simulink model "Lab2_simulink_plc"  (R2023b) (continued)
== system Lab2_simulink_plc/PLC Controller Suite/__Logic/IR logic/IR sensor logic/__Logic == (continued)
      mask init:
      | block = gcb;
      | maskObj = Simulink.Mask.get(block);
      | maskObj.Display = sprintf('disp([''%s'' newline newline ''%s'']);', PLCBlockType, PLCOperandTag);
      | slplc.callbacks.initInstruction(block);
      settings: AttributesFormatString=%<PLCBlockType>: %<PLCOperandTag>
      mask Help: web([docroot '/plccoder/ref/ton' '.html']);
      mask DialogControl PLCBlockTitle Prompt: Timer On Delay (TON)
      mask Display: disp(['TON' newline newline 'medium_on']);
  TON5 : Subsystem  Priority=74  TreatAsAtomicUnit=on  mask{PLCOperandTag=medium_off, PLCTimerSampleTime=0.2, PLCBlockType=TON, PLCPOUType=stdFB, PLCOperandAccess=ReadWrite, PLCOperandExpression=xxx_PLC_VAR_medium_off}
      mask prompts: PLCOperandTag "Timer Tag:"; PLCTimerSampleTime "Timer Sample Time:"
      mask init:
      | block = gcb;
      | maskObj = Simulink.Mask.get(block);
      | maskObj.Display = sprintf('disp([''%s'' newline newline ''%s'']);', PLCBlockType, PLCOperandTag);
      | slplc.callbacks.initInstruction(block);
      settings: AttributesFormatString=%<PLCBlockType>: %<PLCOperandTag>
      mask Help: web([docroot '/plccoder/ref/ton' '.html']);
      mask DialogControl PLCBlockTitle Prompt: Timer On Delay (TON)
      mask Display: disp(['TON' newline newline 'medium_off']);
  Up to Parent POU : Subsystem  DisableCoverage=on  TreatAsAtomicUnit=on  mask{PLCBlockType=OpenParentPOU}
      settings: OpenFcn=slplc.callbacks.openParentPOUBlock(gcb);
      mask Help: web([docroot '/plccoder/ref/uptoparentpou' '.html']);
      mask DialogControl PLCBlockTitle Prompt: Add Single Rung
  Variable Read : Subsystem  Priority=34  TreatAsAtomicUnit=on  mask{PLCOperandTag=IR_value_copy, PLCBlockType=VariableRead, PLCOperandAccess=Read, PLCPOUType=VariableRead, PLCOperandExpression=xxx_PLC_VAR_IR_value_copy}
      mask prompts: PLCOperandTag "Data Tag:"
      mask init:
      | block = gcb;
      | maskObj = Simulink.Mask.get(block);
      | maskObj.Display = sprintf('disp(''%s'');', PLCOperandTag);
      | slplc.callbacks.initInstruction(block);
      mask Help: web([docroot '/plccoder/ref/variableread' '.html']);
      mask DialogControl PLCBlockTitle Prompt: Data Tag Read
      mask Display: disp('IR_value_copy');
  Variable Read1 : Subsystem  Priority=7  TreatAsAtomicUnit=on  mask{PLCOperandTag=IR_value_copy, PLCBlockType=VariableRead, PLCOperandAccess=Read, PLCPOUType=VariableRead, PLCOperandExpression=xxx_PLC_VAR_IR_value_copy}
      mask prompts: PLCOperandTag "Data Tag:"
      mask init:
      | block = gcb;
      | maskObj = Simulink.Mask.get(block);
      | maskObj.Display = sprintf('disp(''%s'');', PLCOperandTag);
      | slplc.callbacks.initInstruction(block);
      mask Help: web([docroot '/plccoder/ref/variableread' '.html']);
      mask DialogControl PLCBlockTitle Prompt: Data Tag Read
      mask Display: disp('IR_value_copy');
  Variable Read10 : Subsystem  Priority=2  TreatAsAtomicUnit=on  mask{PLCOperandTag=IR_value_copy, PLCBlockType=VariableRead, PLCOperandAccess=Read, PLCPOUType=VariableRead, PLCOperandExpression=xxx_PLC_VAR_IR_value_copy}
      mask prompts: PLCOperandTag "Data Tag:"
      mask init:
      | block = gcb;
      | maskObj = Simulink.Mask.get(block);
      | maskObj.Display = sprintf('disp(''%s'');', PLCOperandTag);
      | slplc.callbacks.initInstruction(block);
      mask Help: web([docroot '/plccoder/ref/variableread' '.html']);
      mask DialogControl PLCBlockTitle Prompt: Data Tag Read
      mask Display: disp('IR_value_copy');
  Variable Read11 : Subsystem  Priority=24  TreatAsAtomicUnit=on  mask{PLCOperandTag=half_constant, PLCBlockType=VariableRead, PLCOperandAccess=Read, PLCPOUType=VariableRead, PLCOperandExpression=xxx_PLC_VAR_half_constant}
      mask prompts: PLCOperandTag "Data Tag:"
      mask init:
      | block = gcb;
      | maskObj = Simulink.Mask.get(block);
      | maskObj.Display = sprintf('disp(''%s'');', PLCOperandTag);
      | slplc.callbacks.initInstruction(block);
      mask Help: web([docroot '/plccoder/ref/variableread' '.html']);
      mask DialogControl PLCBlockTitle Prompt: Data Tag Read
      mask Display: disp('half_constant');
  Variable Read12 : Subsystem  Priority=16  TreatAsAtomicUnit=on  mask{PLCOperandTag=IR_value_copy, PLCBlockType=VariableRead, PLCOperandAccess=Read, PLCPOUType=VariableRead, PLCOperandExpression=xxx_PLC_VAR_IR_value_copy}
      mask prompts: PLCOperandTag "Data Tag:"
      mask init:
      | block = gcb;
      | maskObj = Simulink.Mask.get(block);
      | maskObj.Display = sprintf('disp(''%s'');', PLCOperandTag);
      | slplc.callbacks.initInstruction(block);
      mask Help: web([docroot '/plccoder/ref/variableread' '.html']);
      mask DialogControl PLCBlockTitle Prompt: Data Tag Read
      mask Display: disp('IR_value_copy');
  Variable Read13 : Subsystem  Priority=17  TreatAsAtomicUnit=on  mask{PLCOperandTag=close_constant, PLCBlockType=VariableRead, PLCOperandAccess=Read, PLCPOUType=VariableRead, PLCOperandExpression=xxx_PLC_VAR_close_constant}
      mask prompts: PLCOperandTag "Data Tag:"
      mask init:
      | block = gcb;
      | maskObj = Simulink.Mask.get(block);
      | maskObj.Display = sprintf('disp(''%s'');', PLCOperandTag);
      | slplc.callbacks.initInstruction(block);
      mask Help: web([docroot '/plccoder/ref/variableread' '.html']);
      mask DialogControl PLCBlockTitle Prompt: Data Tag Read
      mask Display: disp('close_constant');
  Variable Read14 : Subsystem  Priority=40  TreatAsAtomicUnit=on  mask{PLCOperandTag=duty_cycle, PLCBlockType=VariableRead, PLCOperandAccess=Read, PLCPOUType=VariableRead, PLCOperandExpression=xxx_PLC_VAR_duty_cycle}
      mask prompts: PLCOperandTag "Data Tag:"
      mask init:
      | block = gcb;
      | maskObj = Simulink.Mask.get(block);
      | maskObj.Display = sprintf('disp(''%s'');', PLCOperandTag);
      | slplc.callbacks.initInstruction(block);
      mask Help: web([docroot '/plccoder/ref/variableread' '.html']);
      mask DialogControl PLCBlockTitle Prompt: Data Tag Read
      mask Display: disp('duty_cycle');
  Variable Read15 : Subsystem  Priority=47  TreatAsAtomicUnit=on  mask{PLCOperandTag=duty_cycle, PLCBlockType=VariableRead, PLCOperandAccess=Read, PLCPOUType=VariableRead, PLCOperandExpression=xxx_PLC_VAR_duty_cycle}
      mask prompts: PLCOperandTag "Data Tag:"
      mask init:
      | block = gcb;
      | maskObj = Simulink.Mask.get(block);
      | maskObj.Display = sprintf('disp(''%s'');', PLCOperandTag);
      | slplc.callbacks.initInstruction(block);
      mask Help: web([docroot '/plccoder/ref/variableread' '.html']);
      mask DialogControl PLCBlockTitle Prompt: Data Tag Read
      mask Display: disp('duty_cycle');
  Variable Read16 : Subsystem  Priority=55  TreatAsAtomicUnit=on  mask{PLCOperandTag=green_off, PLCBlockType=VariableRead, PLCOperandAccess=Read, PLCPOUType=VariableRead, PLCOperandExpression=xxx_PLC_VAR_green_off}
      mask prompts: PLCOperandTag "Data Tag:"
      mask init:
      | block = gcb;
      | maskObj = Simulink.Mask.get(block);
      | maskObj.Display = sprintf('disp(''%s'');', PLCOperandTag);
      | slplc.callbacks.initInstruction(block);
      mask Help: web([docroot '/plccoder/ref/variableread' '.html']);
      mask DialogControl PLCBlockTitle Prompt: Data Tag Read
      mask Display: disp('green_off');
  Variable Read17 : Subsystem  Priority=63  TreatAsAtomicUnit=on  mask{PLCOperandTag=duty_cycle, PLCBlockType=VariableRead, PLCOperandAccess=Read, PLCPOUType=VariableRead, PLCOperandExpression=xxx_PLC_VAR_duty_cycle}
      mask prompts: PLCOperandTag "Data Tag:"
      mask init:
      | block = gcb;
      | maskObj = Simulink.Mask.get(block);
      | maskObj.Display = sprintf('disp(''%s'');', PLCOperandTag);
      | slplc.callbacks.initInstruction(block);
      mask Help: web([docroot '/plccoder/ref/variableread' '.html']);
      mask DialogControl PLCBlockTitle Prompt: Data Tag Read
      mask Display: disp('duty_cycle');
  Variable Read18 : Subsystem  Priority=79  TreatAsAtomicUnit=on  mask{PLCOperandTag=duty_cycle, PLCBlockType=VariableRead, PLCOperandAccess=Read, PLCPOUType=VariableRead, PLCOperandExpression=xxx_PLC_VAR_duty_cycle}
      mask prompts: PLCOperandTag "Data Tag:"
      mask init:
      | block = gcb;
      | maskObj = Simulink.Mask.get(block);
      | maskObj.Display = sprintf('disp(''%s'');', PLCOperandTag);
      | slplc.callbacks.initInstruction(block);
      mask Help: web([docroot '/plccoder/ref/variableread' '.html']);
      mask DialogControl PLCBlockTitle Prompt: Data Tag Read
      mask Display: disp('duty_cycle');
  Variable Read19 : Subsystem  Priority=71  TreatAsAtomicUnit=on  mask{PLCOperandTag=green_off, PLCBlockType=VariableRead, PLCOperandAccess=Read, PLCPOUType=VariableRead, PLCOperandExpression=xxx_PLC_VAR_green_off}
      mask prompts: PLCOperandTag "Data Tag:"
      mask init:
      | block = gcb;
      | maskObj = Simulink.Mask.get(block);
      | maskObj.Display = sprintf('disp(''%s'');', PLCOperandTag);
      | slplc.callbacks.initInstruction(block);
      mask Help: web([docroot '/plccoder/ref/variableread' '.html']);
      mask DialogControl PLCBlockTitle Prompt: Data Tag Read
      mask Display: disp('green_off');
  Variable Read2 : Subsystem  Priority=35  TreatAsAtomicUnit=on  mask{PLCOperandTag=far_constant, PLCBlockType=VariableRead, PLCOperandAccess=Read, PLCPOUType=VariableRead, PLCOperandExpression=xxx_PLC_VAR_far_constant}
      mask prompts: PLCOperandTag "Data Tag:"
      mask init:
      | block = gcb;
      | maskObj = Simulink.Mask.get(block);
      | maskObj.Display = sprintf('disp(''%s'');', PLCOperandTag);
      | slplc.callbacks.initInstruction(block);
      mask Help: web([docroot '/plccoder/ref/variableread' '.html']);
      mask DialogControl PLCBlockTitle Prompt: Data Tag Read
      mask Display: disp('far_constant');
  Variable Read20 : Subsystem  Priority=87  TreatAsAtomicUnit=on  mask{PLCOperandTag=green_off, PLCBlockType=VariableRead, PLCOperandAccess=Read, PLCPOUType=VariableRead, PLCOperandExpression=xxx_PLC_VAR_green_off}
      mask prompts: PLCOperandTag "Data Tag:"
      mask init:
      | block = gcb;
      | maskObj = Simulink.Mask.get(block);
      | maskObj.Display = sprintf('disp(''%s'');', PLCOperandTag);
      | slplc.callbacks.initInstruction(block);
      mask Help: web([docroot '/plccoder/ref/variableread' '.html']);
      mask DialogControl PLCBlockTitle Prompt: Data Tag Read
      mask Display: disp('green_off');
  Variable Read3 : Subsystem  Priority=8  TreatAsAtomicUnit=on  mask{PLCOperandTag=scope_constant, PLCBlockType=VariableRead, PLCOperandAccess=Read, PLCPOUType=VariableRead, PLCOperandExpression=xxx_PLC_VAR_scope_constant}
      mask prompts: PLCOperandTag "Data Tag:"
      mask init:
      | block = gcb;
      | maskObj = Simulink.Mask.get(block);
      | maskObj.Display = sprintf('disp(''%s'');', PLCOperandTag);
      | slplc.callbacks.initInstruction(block);
      mask Help: web([docroot '/plccoder/ref/variableread' '.html']);
      mask DialogControl PLCBlockTitle Prompt: Data Tag Read
      mask Display: disp('scope_constant');
  Variable Read4 : Subsystem  Priority=13  TreatAsAtomicUnit=on  mask{PLCOperandTag=IR_value_copy, PLCBlockType=VariableRead, PLCOperandAccess=Read, PLCPOUType=VariableRead, PLCOperandExpression=xxx_PLC_VAR_IR_value_copy}
      mask prompts: PLCOperandTag "Data Tag:"
      mask init:
      | block = gcb;
      | maskObj = Simulink.Mask.get(block);
      | maskObj.Display = sprintf('disp(''%s'');', PLCOperandTag);
      | slplc.callbacks.initInstruction(block);
      mask Help: web([docroot '/plccoder/ref/variableread' '.html']);
      mask DialogControl PLCBlockTitle Prompt: Data Tag Read
      mask Display: disp('IR_value_copy');
  Variable Read5 : Subsystem  Priority=14  TreatAsAtomicUnit=on  mask{PLCOperandTag=half_constant, PLCBlockType=VariableRead, PLCOperandAccess=Read, PLCPOUType=VariableRead, PLCOperandExpression=xxx_PLC_VAR_half_constant}
      mask prompts: PLCOperandTag "Data Tag:"
      mask init:
      | block = gcb;
      | maskObj = Simulink.Mask.get(block);
      | maskObj.Display = sprintf('disp(''%s'');', PLCOperandTag);
      | slplc.callbacks.initInstruction(block);
      mask Help: web([docroot '/plccoder/ref/variableread' '.html']);
      mask DialogControl PLCBlockTitle Prompt: Data Tag Read
      mask Display: disp('half_constant');
  Variable Read6 : Subsystem  Priority=23  TreatAsAtomicUnit=on  mask{PLCOperandTag=IR_value_copy, PLCBlockType=VariableRead, PLCOperandAccess=Read, PLCPOUType=VariableRead, PLCOperandExpression=xxx_PLC_VAR_IR_value_copy}
      mask prompts: PLCOperandTag "Data Tag:"
      mask init:
      | block = gcb;
      | maskObj = Simulink.Mask.get(block);
      | maskObj.Display = sprintf('disp(''%s'');', PLCOperandTag);
      | slplc.callbacks.initInstruction(block);
      mask Help: web([docroot '/plccoder/ref/variableread' '.html']);
      mask DialogControl PLCBlockTitle Prompt: Data Tag Read
      mask Display: disp('IR_value_copy');
  Variable Read7 : Subsystem  Priority=26  TreatAsAtomicUnit=on  mask{PLCOperandTag=IR_value_copy, PLCBlockType=VariableRead, PLCOperandAccess=Read, PLCPOUType=VariableRead, PLCOperandExpression=xxx_PLC_VAR_IR_value_copy}
      mask prompts: PLCOperandTag "Data Tag:"
      mask init:
      | block = gcb;
      | maskObj = Simulink.Mask.get(block);
      | maskObj.Display = sprintf('disp(''%s'');', PLCOperandTag);
      | slplc.callbacks.initInstruction(block);
      mask Help: web([docroot '/plccoder/ref/variableread' '.html']);
      mask DialogControl PLCBlockTitle Prompt: Data Tag Read
      mask Display: disp('IR_value_copy');
  Variable Read8 : Subsystem  Priority=27  TreatAsAtomicUnit=on  mask{PLCOperandTag=far_constant, PLCBlockType=VariableRead, PLCOperandAccess=Read, PLCPOUType=VariableRead, PLCOperandExpression=xxx_PLC_VAR_far_constant}
      mask prompts: PLCOperandTag "Data Tag:"
      mask init:
      | block = gcb;
      | maskObj = Simulink.Mask.get(block);
      | maskObj.Display = sprintf('disp(''%s'');', PLCOperandTag);
      | slplc.callbacks.initInstruction(block);
      mask Help: web([docroot '/plccoder/ref/variableread' '.html']);
      mask DialogControl PLCBlockTitle Prompt: Data Tag Read
      mask Display: disp('far_constant');
  Variable Read9 : Subsystem  Priority=3  TreatAsAtomicUnit=on  mask{PLCOperandTag=constant, PLCBlockType=VariableRead, PLCOperandAccess=Read, PLCPOUType=VariableRead, PLCOperandExpression=xxx_PLC_VAR_constant}
      mask prompts: PLCOperandTag "Data Tag:"
      mask init:
      | block = gcb;
      | maskObj = Simulink.Mask.get(block);
      | maskObj.Display = sprintf('disp(''%s'');', PLCOperandTag);
      | slplc.callbacks.initInstruction(block);
      mask Help: web([docroot '/plccoder/ref/variableread' '.html']);
      mask DialogControl PLCBlockTitle Prompt: Data Tag Read
      mask Display: disp('constant');
  Variable Write : Subsystem  Priority=5  TreatAsAtomicUnit=on  mask{PLCOperandTag=duty_cycle, PLCBlockType=VariableWrite, PLCOperandAccess=Write, PLCPOUType=VariableWrite, PLCOperandExpression=xxx_PLC_VAR_duty_cycle}
      mask prompts: PLCOperandTag "Data Tag:"
      mask init: same as Lab2_simulink_plc/PLC Controller Suite/__Logic/Fukt logic/Program/__Logic/Variable Write
      mask Help: web([docroot '/plccoder/ref/variablewrite' '.html']);
      mask DialogControl PLCBlockTitle Prompt: Data Tag Write
      mask Display: disp('duty_cycle');
  Variable Write1 : Subsystem  Priority=42  TreatAsAtomicUnit=on  mask{PLCOperandTag=GreenLed, PLCBlockType=VariableWrite, PLCOperandAccess=Write, PLCPOUType=VariableWrite, PLCOperandExpression=xxx_PLC_VAR_GreenLed}
      mask prompts: PLCOperandTag "Data Tag:"
      mask init: same as Lab2_simulink_plc/PLC Controller Suite/__Logic/Fukt logic/Program/__Logic/Variable Write
      mask Help: web([docroot '/plccoder/ref/variablewrite' '.html']);
      mask DialogControl PLCBlockTitle Prompt: Data Tag Write
      mask Display: disp('GreenLed');
  Variable Write2 : Subsystem  Priority=49  TreatAsAtomicUnit=on  mask{PLCOperandTag=GreenLed, PLCBlockType=VariableWrite, PLCOperandAccess=Write, PLCPOUType=VariableWrite, PLCOperandExpression=xxx_PLC_VAR_GreenLed}
      mask prompts: PLCOperandTag "Data Tag:"
      mask init: same as Lab2_simulink_plc/PLC Controller Suite/__Logic/Fukt logic/Program/__Logic/Variable Write
      mask Help: web([docroot '/plccoder/ref/variablewrite' '.html']);
      mask DialogControl PLCBlockTitle Prompt: Data Tag Write
      mask Display: disp('GreenLed');
  Variable Write3 : Subsystem  Priority=57  TreatAsAtomicUnit=on  mask{PLCOperandTag=GreenLed, PLCBlockType=VariableWrite, PLCOperandAccess=Write, PLCPOUType=VariableWrite, PLCOperandExpression=xxx_PLC_VAR_GreenLed}
      mask prompts: PLCOperandTag "Data Tag:"
      mask init: same as Lab2_simulink_plc/PLC Controller Suite/__Logic/Fukt logic/Program/__Logic/Variable Write
      mask Help: web([docroot '/plccoder/ref/variablewrite' '.html']);
      mask DialogControl PLCBlockTitle Prompt: Data Tag Write
      mask Display: disp('GreenLed');
  Variable Write4 : Subsystem  Priority=65  TreatAsAtomicUnit=on  mask{PLCOperandTag=GreenLed, PLCBlockType=VariableWrite, PLCOperandAccess=Write, PLCPOUType=VariableWrite, PLCOperandExpression=xxx_PLC_VAR_GreenLed}
      mask prompts: PLCOperandTag "Data Tag:"
      mask init: same as Lab2_simulink_plc/PLC Controller Suite/__Logic/Fukt logic/Program/__Logic/Variable Write
      mask Help: web([docroot '/plccoder/ref/variablewrite' '.html']);
      mask DialogControl PLCBlockTitle Prompt: Data Tag Write
      mask Display: disp('GreenLed');
  Variable Write5 : Subsystem  Priority=81  TreatAsAtomicUnit=on  mask{PLCOperandTag=GreenLed, PLCBlockType=VariableWrite, PLCOperandAccess=Write, PLCPOUType=VariableWrite, PLCOperandExpression=xxx_PLC_VAR_GreenLed}
      mask prompts: PLCOperandTag "Data Tag:"
      mask init: same as Lab2_simulink_plc/PLC Controller Suite/__Logic/Fukt logic/Program/__Logic/Variable Write
      mask Help: web([docroot '/plccoder/ref/variablewrite' '.html']);
      mask DialogControl PLCBlockTitle Prompt: Data Tag Write
      mask Display: disp('GreenLed');
  Variable Write6 : Subsystem  Priority=73  TreatAsAtomicUnit=on  mask{PLCOperandTag=GreenLed, PLCBlockType=VariableWrite, PLCOperandAccess=Write, PLCPOUType=VariableWrite, PLCOperandExpression=xxx_PLC_VAR_GreenLed}
      mask prompts: PLCOperandTag "Data Tag:"
      mask init: same as Lab2_simulink_plc/PLC Controller Suite/__Logic/Fukt logic/Program/__Logic/Variable Write
      mask Help: web([docroot '/plccoder/ref/variablewrite' '.html']);
      mask DialogControl PLCBlockTitle Prompt: Data Tag Write
      mask Display: disp('GreenLed');
  Variable Write7 : Subsystem  Priority=89  TreatAsAtomicUnit=on  mask{PLCOperandTag=GreenLed, PLCBlockType=VariableWrite, PLCOperandAccess=Write, PLCPOUType=VariableWrite, PLCOperandExpression=xxx_PLC_VAR_GreenLed}
      mask prompts: PLCOperandTag "Data Tag:"
      mask init: same as Lab2_simulink_plc/PLC Controller Suite/__Logic/Fukt logic/Program/__Logic/Variable Write
      mask Help: web([docroot '/plccoder/ref/variablewrite' '.html']);
      mask DialogControl PLCBlockTitle Prompt: Data Tag Write
      mask Display: disp('GreenLed');
  XIC : Subsystem  Priority=86  TreatAsAtomicUnit=on  mask{PLCOperandTag=slow_on.DN, PLCBlockType=XIC, PLCPOUType=Contact, PLCOperandExpression=xxx_PLC_VAR_slow_on.DN}
      mask prompts: PLCOperandTag "Operand Tag:"
      mask init:
      | slplc.callbacks.initInstruction(gcb);
      settings: AttributesFormatString=%<PLCOperandTag>
      mask Help: web([docroot '/plccoder/ref/xic' '.html']);
      mask DialogControl PLCBlockTitle Prompt: Examine If Closed (XIC)
  XIC1 : Subsystem  Priority=39  TreatAsAtomicUnit=on  mask{PLCOperandTag=out_of_scope, PLCBlockType=XIC, PLCPOUType=Contact, PLCOperandExpression=xxx_PLC_VAR_out_of_scope}
      mask prompts: PLCOperandTag "Operand Tag:"
      mask init:
      | slplc.callbacks.initInstruction(gcb);
      settings: AttributesFormatString=%<PLCOperandTag>
      mask Help: web([docroot '/plccoder/ref/xic' '.html']);
      mask DialogControl PLCBlockTitle Prompt: Examine If Closed (XIC)
  XIC2 : Subsystem  Priority=53  TreatAsAtomicUnit=on  mask{PLCOperandTag=close, PLCBlockType=XIC, PLCPOUType=Contact, PLCOperandExpression=xxx_PLC_VAR_close}
      mask prompts: PLCOperandTag "Operand Tag:"
      mask init:
      | slplc.callbacks.initInstruction(gcb);
      settings: AttributesFormatString=%<PLCOperandTag>
      mask Help: web([docroot '/plccoder/ref/xic' '.html']);
      mask DialogControl PLCBlockTitle Prompt: Examine If Closed (XIC)
  XIC3 : Subsystem  Priority=61  TreatAsAtomicUnit=on  mask{PLCOperandTag=half, PLCBlockType=XIC, PLCPOUType=Contact, PLCOperandExpression=xxx_PLC_VAR_half}
      mask prompts: PLCOperandTag "Operand Tag:"
      mask init:
      | slplc.callbacks.initInstruction(gcb);
      settings: AttributesFormatString=%<PLCOperandTag>
      mask Help: web([docroot '/plccoder/ref/xic' '.html']);
      mask DialogControl PLCBlockTitle Prompt: Examine If Closed (XIC)
  XIC4 : Subsystem  Priority=54  TreatAsAtomicUnit=on  mask{PLCOperandTag=fast_on.DN, PLCBlockType=XIC, PLCPOUType=Contact, PLCOperandExpression=xxx_PLC_VAR_fast_on.DN}
      mask prompts: PLCOperandTag "Operand Tag:"
      mask init:
      | slplc.callbacks.initInstruction(gcb);
      settings: AttributesFormatString=%<PLCOperandTag>
      mask Help: web([docroot '/plccoder/ref/xic' '.html']);
      mask DialogControl PLCBlockTitle Prompt: Examine If Closed (XIC)
  XIC5 : Subsystem  Priority=70  TreatAsAtomicUnit=on  mask{PLCOperandTag=medium_on.DN, PLCBlockType=XIC, PLCPOUType=Contact, PLCOperandExpression=xxx_PLC_VAR_medium_on.DN}
      mask prompts: PLCOperandTag "Operand Tag:"
      mask init:
      | slplc.callbacks.initInstruction(gcb);
      settings: AttributesFormatString=%<PLCOperandTag>
      mask Help: web([docroot '/plccoder/ref/xic' '.html']);
      mask DialogControl PLCBlockTitle Prompt: Examine If Closed (XIC)
  XIC6 : Subsystem  Priority=45  TreatAsAtomicUnit=on  mask{PLCOperandTag=close, PLCBlockType=XIC, PLCPOUType=Contact, PLCOperandExpression=xxx_PLC_VAR_close}
      mask prompts: PLCOperandTag "Operand Tag:"
      mask init:
      | slplc.callbacks.initInstruction(gcb);
      settings: AttributesFormatString=%<PLCOperandTag>
      mask Help: web([docroot '/plccoder/ref/xic' '.html']);
      mask DialogControl PLCBlockTitle Prompt: Examine If Closed (XIC)
  XIC7 : Subsystem  Priority=69  TreatAsAtomicUnit=on  mask{PLCOperandTag=half, PLCBlockType=XIC, PLCPOUType=Contact, PLCOperandExpression=xxx_PLC_VAR_half}
      mask prompts: PLCOperandTag "Operand Tag:"
      mask init:
      | slplc.callbacks.initInstruction(gcb);
      settings: AttributesFormatString=%<PLCOperandTag>
      mask Help: web([docroot '/plccoder/ref/xic' '.html']);
      mask DialogControl PLCBlockTitle Prompt: Examine If Closed (XIC)
  XIC8 : Subsystem  Priority=77  TreatAsAtomicUnit=on  mask{PLCOperandTag=far, PLCBlockType=XIC, PLCPOUType=Contact, PLCOperandExpression=xxx_PLC_VAR_far}
      mask prompts: PLCOperandTag "Operand Tag:"
      mask init:
      | slplc.callbacks.initInstruction(gcb);
      settings: AttributesFormatString=%<PLCOperandTag>
      mask Help: web([docroot '/plccoder/ref/xic' '.html']);
      mask DialogControl PLCBlockTitle Prompt: Examine If Closed (XIC)
  XIC9 : Subsystem  Priority=85  TreatAsAtomicUnit=on  mask{PLCOperandTag=far, PLCBlockType=XIC, PLCPOUType=Contact, PLCOperandExpression=xxx_PLC_VAR_far}
      mask prompts: PLCOperandTag "Operand Tag:"
      mask init:
      | slplc.callbacks.initInstruction(gcb);
      settings: AttributesFormatString=%<PLCOperandTag>
      mask Help: web([docroot '/plccoder/ref/xic' '.html']);
      mask DialogControl PLCBlockTitle Prompt: Examine If Closed (XIC)
  XIO : Subsystem  Priority=78  TreatAsAtomicUnit=on  mask{PLCOperandExpression=xxx_PLC_VAR_slow_off.DN, PLCOperandTag=slow_off.DN, PLCBlockType=XIO, PLCPOUType=Contact}
      mask prompts: PLCOperandTag "Operand Tag:"
      mask init:
      | slplc.callbacks.initInstruction(gcb);
      settings: AttributesFormatString=%<PLCOperandTag>
      mask Help: web([docroot '/plccoder/ref/xio' '.html']);
      mask DialogControl PLCBlockTitle Prompt: Examine If Open (XIO)
  XIO1 : Subsystem  Priority=31  TreatAsAtomicUnit=on  mask{PLCOperandExpression=xxx_PLC_VAR_out_of_scope, PLCOperandTag=out_of_scope, PLCBlockType=XIO, PLCPOUType=Contact}
      mask prompts: PLCOperandTag "Operand Tag:"
      mask init:
      | slplc.callbacks.initInstruction(gcb);
      settings: AttributesFormatString=%<PLCOperandTag>
      mask Help: web([docroot '/plccoder/ref/xio' '.html']);
      mask DialogControl PLCBlockTitle Prompt: Examine If Open (XIO)
  XIO10 : Subsystem  Priority=76  TreatAsAtomicUnit=on  mask{PLCOperandExpression=xxx_PLC_VAR_out_of_scope, PLCOperandTag=out_of_scope, PLCBlockType=XIO, PLCPOUType=Contact}
      mask prompts: PLCOperandTag "Operand Tag:"
      mask init:
      | slplc.callbacks.initInstruction(gcb);
      settings: AttributesFormatString=%<PLCOperandTag>
      mask Help: web([docroot '/plccoder/ref/xio' '.html']);
      mask DialogControl PLCBlockTitle Prompt: Examine If Open (XIO)
  XIO11 : Subsystem  Priority=22  TreatAsAtomicUnit=on  mask{PLCOperandExpression=xxx_PLC_VAR_out_of_scope, PLCOperandTag=out_of_scope, PLCBlockType=XIO, PLCPOUType=Contact}
      mask prompts: PLCOperandTag "Operand Tag:"
      mask init:
      | slplc.callbacks.initInstruction(gcb);
      settings: AttributesFormatString=%<PLCOperandTag>
      mask Help: web([docroot '/plccoder/ref/xio' '.html']);
      mask DialogControl PLCBlockTitle Prompt: Examine If Open (XIO)
  XIO12 : Subsystem  Priority=21  TreatAsAtomicUnit=on  mask{PLCOperandExpression=xxx_PLC_VAR_close, PLCOperandTag=close, PLCBlockType=XIO, PLCPOUType=Contact}
      mask prompts: PLCOperandTag "Operand Tag:"
      mask init:
      | slplc.callbacks.initInstruction(gcb);
      settings: AttributesFormatString=%<PLCOperandTag>
      mask Help: web([docroot '/plccoder/ref/xio' '.html']);
      mask DialogControl PLCBlockTitle Prompt: Examine If Open (XIO)
  XIO13 : Subsystem  Priority=32  TreatAsAtomicUnit=on  mask{PLCOperandExpression=xxx_PLC_VAR_close, PLCOperandTag=close, PLCBlockType=XIO, PLCPOUType=Contact}
      mask prompts: PLCOperandTag "Operand Tag:"
      mask init:
      | slplc.callbacks.initInstruction(gcb);
      settings: AttributesFormatString=%<PLCOperandTag>
      mask Help: web([docroot '/plccoder/ref/xio' '.html']);
      mask DialogControl PLCBlockTitle Prompt: Examine If Open (XIO)
  XIO14 : Subsystem  Priority=33  TreatAsAtomicUnit=on  mask{PLCOperandExpression=xxx_PLC_VAR_half, PLCOperandTag=half, PLCBlockType=XIO, PLCPOUType=Contact}
      mask prompts: PLCOperandTag "Operand Tag:"
      mask init:
      | slplc.callbacks.initInstruction(gcb);
      settings: AttributesFormatString=%<PLCOperandTag>
      mask Help: web([docroot '/plccoder/ref/xio' '.html']);
      mask DialogControl PLCBlockTitle Prompt: Examine If Open (XIO)
  XIO2 : Subsystem  Priority=12  TreatAsAtomicUnit=on  mask{PLCOperandExpression=xxx_PLC_VAR_out_of_scope, PLCOperandTag=out_of_scope, PLCBlockType=XIO, PLCPOUType=Contact}
      mask prompts: PLCOperandTag "Operand Tag:"
      mask init:
      | slplc.callbacks.initInstruction(gcb);
      settings: AttributesFormatString=%<PLCOperandTag>
      mask Help: web([docroot '/plccoder/ref/xio' '.html']);
      mask DialogControl PLCBlockTitle Prompt: Examine If Open (XIO)
  XIO3 : Subsystem  Priority=52  TreatAsAtomicUnit=on  mask{PLCOperandExpression=xxx_PLC_VAR_out_of_scope, PLCOperandTag=out_of_scope, PLCBlockType=XIO, PLCPOUType=Contact}
      mask prompts: PLCOperandTag "Operand Tag:"
      mask init:
      | slplc.callbacks.initInstruction(gcb);
      settings: AttributesFormatString=%<PLCOperandTag>
      mask Help: web([docroot '/plccoder/ref/xio' '.html']);
      mask DialogControl PLCBlockTitle Prompt: Examine If Open (XIO)
  XIO4 : Subsystem  Priority=60  TreatAsAtomicUnit=on  mask{PLCOperandExpression=xxx_PLC_VAR_out_of_scope, PLCOperandTag=out_of_scope, PLCBlockType=XIO, PLCPOUType=Contact}
      mask prompts: PLCOperandTag "Operand Tag:"
      mask init:
      | slplc.callbacks.initInstruction(gcb);
      settings: AttributesFormatString=%<PLCOperandTag>
      mask Help: web([docroot '/plccoder/ref/xio' '.html']);
      mask DialogControl PLCBlockTitle Prompt: Examine If Open (XIO)
  XIO5 : Subsystem  Priority=84  TreatAsAtomicUnit=on  mask{PLCOperandExpression=xxx_PLC_VAR_out_of_scope, PLCOperandTag=out_of_scope, PLCBlockType=XIO, PLCPOUType=Contact}
      mask prompts: PLCOperandTag "Operand Tag:"
      mask init:
      | slplc.callbacks.initInstruction(gcb);
      settings: AttributesFormatString=%<PLCOperandTag>
      mask Help: web([docroot '/plccoder/ref/xio' '.html']);
      mask DialogControl PLCBlockTitle Prompt: Examine If Open (XIO)
  XIO6 : Subsystem  Priority=46  TreatAsAtomicUnit=on  mask{PLCOperandExpression=xxx_PLC_VAR_fast_off.DN, PLCOperandTag=fast_off.DN, PLCBlockType=XIO, PLCPOUType=Contact}
      mask prompts: PLCOperandTag "Operand Tag:"
      mask init:
      | slplc.callbacks.initInstruction(gcb);
      settings: AttributesFormatString=%<PLCOperandTag>
      mask Help: web([docroot '/plccoder/ref/xio' '.html']);
      mask DialogControl PLCBlockTitle Prompt: Examine If Open (XIO)
  XIO7 : Subsystem  Priority=62  TreatAsAtomicUnit=on  mask{PLCOperandExpression=xxx_PLC_VAR_medium_off.DN, PLCOperandTag=medium_off.DN, PLCBlockType=XIO, PLCPOUType=Contact}
      mask prompts: PLCOperandTag "Operand Tag:"
      mask init:
      | slplc.callbacks.initInstruction(gcb);
      settings: AttributesFormatString=%<PLCOperandTag>
      mask Help: web([docroot '/plccoder/ref/xio' '.html']);
      mask DialogControl PLCBlockTitle Prompt: Examine If Open (XIO)
  XIO8 : Subsystem  Priority=44  TreatAsAtomicUnit=on  mask{PLCOperandExpression=xxx_PLC_VAR_out_of_scope, PLCOperandTag=out_of_scope, PLCBlockType=XIO, PLCPOUType=Contact}
      mask prompts: PLCOperandTag "Operand Tag:"
      mask init:
      | slplc.callbacks.initInstruction(gcb);
      settings: AttributesFormatString=%<PLCOperandTag>
      mask Help: web([docroot '/plccoder/ref/xio' '.html']);
      mask DialogControl PLCBlockTitle Prompt: Examine If Open (XIO)
  XIO9 : Subsystem  Priority=68  TreatAsAtomicUnit=on  mask{PLCOperandExpression=xxx_PLC_VAR_out_of_scope, PLCOperandTag=out_of_scope, PLCBlockType=XIO, PLCPOUType=Contact}
      mask prompts: PLCOperandTag "Operand Tag:"
      mask init:
      | slplc.callbacks.initInstruction(gcb);
      settings: AttributesFormatString=%<PLCOperandTag>
      mask Help: web([docroot '/plccoder/ref/xio' '.html']);
      mask DialogControl PLCBlockTitle Prompt: Examine If Open (XIO)
  wires:
  TON/1(EN) -> Rung Terminal1/1(RungIn)
  XIO/1(RungOut) -> MOV4/1(RungIn)
  XIC/1(RungOut) -> MOV6/1(RungIn)
  Variable Read/1(VarOut) -> LEQ2/2(srcA)
  Variable Read2/1(VarOut) -> LEQ2/3(srcB)
  Variable Read1/1(VarOut) -> LEQ/2(srcA)
  Variable Read3/1(VarOut) -> LEQ/3(srcB)
  LEQ/1(RungOut) -> OTE/1(RungIn)
  OTE/1(RungOut) -> Rung Terminal2/1(RungIn)
  XIO1/1(RungOut) -> XIO13/1(RungIn)
  Variable Read5/1(VarOut) -> GEQ1/3(srcB)
  Variable Read4/1(VarOut) -> GEQ1/2(srcA)
  OTE2/1(RungOut) -> Rung Terminal4/1(RungIn)
  XIO6/1(RungOut) -> MOV1/1(RungIn)
  TON2/1(EN) -> Rung Terminal7/1(RungIn)
  TON4/1(EN) -> Rung Terminal9/1(RungIn)
  MOV3/1(RungOut) -> TON4/1(RungIn)
  XIC5/1(RungOut) -> MOV5/1(RungIn)
  XIC6/1(RungOut) -> XIO6/1(RungIn)
  XIC2/1(RungOut) -> XIC4/1(RungIn)
  XIO8/1(RungOut) -> XIC6/1(RungIn)
  XIO3/1(RungOut) -> XIC2/1(RungIn)
  XIO4/1(RungOut) -> XIC3/1(RungIn)
  XIO9/1(RungOut) -> XIC7/1(RungIn)
  XIO5/1(RungOut) -> XIC9/1(RungIn)
  XIO10/1(RungOut) -> XIC8/1(RungIn)
  XIC7/1(RungOut) -> XIC5/1(RungIn)
  XIC3/1(RungOut) -> XIO7/1(RungIn)
  XIO2/1(RungOut) -> GEQ1/1(RungIn)
  LEQ2/1(RungOut) -> OTE2/1(RungIn)
  TON3/1(EN) -> Rung Terminal6/1(RungIn)
  TON5/1(EN) -> Rung Terminal8/1(RungIn)
  TON1/1(EN) -> Rung Terminal/1(RungIn)
  XIO12/1(RungOut) -> XIO11/1(RungIn)
  Variable Read6/1(VarOut) -> LEQ1/2(srcA)
  Variable Read11/1(VarOut) -> LEQ1/3(srcB)
  XIO11/1(RungOut) -> LEQ1/1(RungIn)
  OTE3/1(RungOut) -> Rung Terminal10/1(RungIn)
  LEQ1/1(RungOut) -> GRT/1(RungIn)
  GRT/1(RungOut) -> OTE3/1(RungIn)
  Variable Read7/1(VarOut) -> GRT/2(srcA)
  Variable Read8/1(VarOut) -> GRT/3(srcB)
  GEQ1/1(RungOut) -> LEQ4/1(RungIn)
  Variable Read12/1(VarOut) -> LEQ4/2(srcA)
  Variable Read13/1(VarOut) -> LEQ4/3(srcB)
  LEQ4/1(RungOut) -> OTE1/1(RungIn)
  XIO13/1(RungOut) -> XIO14/1(RungIn)
  XIO14/1(RungOut) -> LEQ2/1(RungIn)
  XIC8/1(RungOut) -> XIO/1(RungIn)
  XIC9/1(RungOut) -> XIC/1(RungIn)
  Variable Read10/1(VarOut) -> DIV/2(srcA)
  Variable Read9/1(VarOut) -> DIV/3(srcB)
  DIV/2(dest) -> Variable Write/1(VarIn)
  DIV/1(RungOut) -> Rung Terminal11/1(RungIn)
  XIC1/1(RungOut) -> MOV/1(RungIn)
  MOV/1(RungOut) -> Rung Terminal5/1(RungIn)
  MOV/2(dest) -> Variable Write1/1(VarIn)
  Variable Read14/1(VarOut) -> MOV/2(src)
  Variable Read15/1(VarOut) -> MOV1/2(src)
  MOV1/2(dest) -> Variable Write2/1(VarIn)
  MOV1/1(RungOut) -> TON2/1(RungIn)
  MOV2/2(dest) -> Variable Write3/1(VarIn)
  Variable Read16/1(VarOut) -> MOV2/2(src)
  MOV2/1(RungOut) -> TON3/1(RungIn)
  XIC4/1(RungOut) -> MOV2/1(RungIn)
  MOV3/2(dest) -> Variable Write4/1(VarIn)
  Variable Read17/1(VarOut) -> MOV3/2(src)
  Variable Read18/1(VarOut) -> MOV4/2(src)
  MOV4/2(dest) -> Variable Write5/1(VarIn)
  Variable Read19/1(VarOut) -> MOV5/2(src)
  MOV5/2(dest) -> Variable Write6/1(VarIn)
  MOV6/2(dest) -> Variable Write7/1(VarIn)
  Variable Read20/1(VarOut) -> MOV6/2(src)
  XIO7/1(RungOut) -> MOV3/1(RungIn)
  MOV5/1(RungOut) -> TON5/1(RungIn)
  MOV4/1(RungOut) -> TON/1(RungIn)
  MOV6/1(RungOut) -> TON1/1(RungIn)
  OTE1/1(RungOut) -> Rung Terminal3/1(RungIn)
  Power Rail Start/1(PowerOut) -> XIO12/1(RungIn), XIO1/1(RungIn), XIC1/1(RungIn), XIO8/1(RungIn), Power Rail Terminal/1(PowerIn), XIO5/1(RungIn), XIO10/1(RungIn), XIO9/1(RungIn), XIO4/1(RungIn), XIO3/1(RungIn), XIO2/1(RungIn), LEQ/1(RungIn), DIV/1(RungIn)
